simulink model "HvacActrPosnReqFrnt"  (R2022b) (continued)
== system HvacActrPosnReqFrnt == (continued)
  AirFlapFrntStsForRowFirstForActrVentSideLe_AirFlapFrntStsForRowFirstForActrVentSideLe/1 -> HvacActrPosnReqFrnt_Runnable_sys/12(AirFlapFrntStsForRowFirstForActrVentSideLe_AirFlapFrntStsForRowFirstForActrVentSideLe_read)
  AirFlapFrntStsForRowFirstForActrVentSideRi_AirFlapFrntStsForRowFirstForActrVentSideRi/1 -> HvacActrPosnReqFrnt_Runnable_sys/13(AirFlapFrntStsForRowFirstForActrVentSideRi_AirFlapFrntStsForRowFirstForActrVentSideRi_read)
  AirFlapFrntStsForRowFirstForOsa_AirFlapFrntStsForRowFirstForOsa/1 -> HvacActrPosnReqFrnt_Runnable_sys/14(AirFlapFrntStsForRowFirstForOsa_AirFlapFrntStsForRowFirstForOsa_read)
  AirFlapFrntStsForRowFirstForOsaAndRecirc_AirFlapFrntStsForRowFirstForOsaAndRecirc/1 -> HvacActrPosnReqFrnt_Runnable_sys/15(AirFlapFrntStsForRowFirstForOsaAndRecirc_AirFlapFrntStsForRowFirstForOsaAndRecirc_read)
  AirFlapFrntStsForRowFirstForRecirc_AirFlapFrntStsForRowFirstForRecirc/1 -> HvacActrPosnReqFrnt_Runnable_sys/16(AirFlapFrntStsForRowFirstForRecirc_AirFlapFrntStsForRowFirstForRecirc_read)
  AirFlapFrntStsForRowSecForActrFlr_AirFlapFrntStsForRowSecForActrFlr/1 -> HvacActrPosnReqFrnt_Runnable_sys/17(AirFlapFrntStsForRowSecForActrFlr_AirFlapFrntStsForRowSecForActrFlr_read)
  AirFlapFrntStsForRowSecForActrVent_AirFlapFrntStsForRowSecForActrVent/1 -> HvacActrPosnReqFrnt_Runnable_sys/18(AirFlapFrntStsForRowSecForActrVent_AirFlapFrntStsForRowSecForActrVent_read)
  AirFlapPosnFrntActForRowFirstForRecirc_AirFlapPosnFrntActForRowFirstForRecirc/1 -> HvacActrPosnReqFrnt_Runnable_sys/19(AirFlapPosnFrntActForRowFirstForRecirc_AirFlapPosnFrntActForRowFirstForRecirc_read)
  AirFlapSpdActForAirDistbn_AirFlapSpdActForAirDistbn/1 -> HvacActrPosnReqFrnt_Runnable_sys/20(AirFlapSpdActForAirDistbn_AirFlapSpdActForAirDistbn_read)
  AirFlapSpdActForRecirc_AirFlapSpdActForRecirc/1 -> HvacActrPosnReqFrnt_Runnable_sys/21(AirFlapSpdActForRecirc_AirFlapSpdActForRecirc_read)
  AirFlapSpdActForTFrnt_AirFlapSpdActForTFrnt/1 -> HvacActrPosnReqFrnt_Runnable_sys/22(AirFlapSpdActForTFrnt_AirFlapSpdActForTFrnt_read)
  AmbTFildForClimaNgcc_AmbTFildForClima/1 -> HvacActrPosnReqFrnt_Runnable_sys/23(AmbTFildForClimaNgcc_AmbTFildForClima_read)
  AmprFanBoostVentnCnsReIntLe_AmprFanBoostVentnCnsReIntLe/1 -> HvacActrPosnReqFrnt_Runnable_sys/24(AmprFanBoostVentnCnsReIntLe_AmprFanBoostVentnCnsReIntLe_read)
  AmprFanBoostVentnCnsReIntRi_AmprFanBoostVentnCnsReIntRi/1 -> HvacActrPosnReqFrnt_Runnable_sys/25(AmprFanBoostVentnCnsReIntRi_AmprFanBoostVentnCnsReIntRi_read)
  BlwAftRunReq_BlwAftRunReq/1 -> HvacActrPosnReqFrnt_Runnable_sys/26(BlwAftRunReq_BlwAftRunReq_read)
  BlwAftRunSts_BlwAftRunSts/1 -> HvacActrPosnReqFrnt_Runnable_sys/27(BlwAftRunSts_BlwAftRunSts_read)
  ClimaCfgForActrModVrnt1_ClimaCfgForActrInd1/1 -> HvacActrPosnReqFrnt_Runnable_sys/28(ClimaCfgForActrModVrnt1_ClimaCfgForActrInd1_read)
  ClimaCfgForActrModVrnt1_ClimaCfgForActrModVrnt1/1 -> HvacActrPosnReqFrnt_Runnable_sys/29(ClimaCfgForActrModVrnt1_ClimaCfgForActrModVrnt1_read)
  ClimaCfgForActrModVrnt1_ClimaCfgForActrModVrntCen/1 -> HvacActrPosnReqFrnt_Runnable_sys/30(ClimaCfgForActrModVrnt1_ClimaCfgForActrModVrntCen_read)
  ClimaCfgForActrModVrnt2_ClimaCfgForActrInd2/1 -> HvacActrPosnReqFrnt_Runnable_sys/31(ClimaCfgForActrModVrnt2_ClimaCfgForActrInd2_read)
  ClimaCfgForActrModVrnt2_ClimaCfgForActrModVrnt2/1 -> HvacActrPosnReqFrnt_Runnable_sys/32(ClimaCfgForActrModVrnt2_ClimaCfgForActrModVrnt2_read)
  ClimaCfgForActrRecircAndOsaCmnPrsnt1_ClimaCfgForActrRecircAndOsaCmnPrsnt1/1 -> HvacActrPosnReqFrnt_Runnable_sys/33(ClimaCfgForActrRecircAndOsaCmnPrsnt1_ClimaCfgForActrRecircAndOsaCmnPrsnt1_read)
  ClimaCfgForBlowrInRowSecVentSidePrsnt1_ClimaCfgForBlowrInRowSecVentSidePrsnt1/1 -> HvacActrPosnReqFrnt_Runnable_sys/34(ClimaCfgForBlowrInRowSecVentSidePrsnt1_ClimaCfgForBlowrInRowSecVentSidePrsnt1_read)
  ClimaCfgForCarTyp1_ClimaCfgForCarTyp1/1 -> HvacActrPosnReqFrnt_Runnable_sys/35(ClimaCfgForCarTyp1_ClimaCfgForCarTyp1_read)
  ClimaCfgForClimaCtrlMan1_ClimaCfgForClimaCtrlMan1/1 -> HvacActrPosnReqFrnt_Runnable_sys/36(ClimaCfgForClimaCtrlMan1_ClimaCfgForClimaCtrlMan1_read)
  ClimaCfgForExtVentnClimaCtrl_ClimaCfgForExtVentnClimaCtrl/1 -> HvacActrPosnReqFrnt_Runnable_sys/37(ClimaCfgForExtVentnClimaCtrl_ClimaCfgForExtVentnClimaCtrl_read)
  ClimaCfgForFanPotiCtrld1_ClimaCfgForFanPotiCtrld1/1 -> HvacActrPosnReqFrnt_Runnable_sys/38(ClimaCfgForFanPotiCtrld1_ClimaCfgForFanPotiCtrld1_read)
  ClimaCfgForHvacFrontBlowerType_ClimaCfgForHvacFrontBlowerType/1 -> HvacActrPosnReqFrnt_Runnable_sys/39(ClimaCfgForHvacFrontBlowerType_ClimaCfgForHvacFrontBlowerType_read)
  ClimaCfgForVehElec1_ClimaCfgForVehElec1/1 -> HvacActrPosnReqFrnt_Runnable_sys/40(ClimaCfgForVehElec1_ClimaCfgForVehElec1_read)
  ClimaCfgForVrntOfVehSysElec1_ClimaCfgForVrntOfVehSysElec1/1 -> HvacActrPosnReqFrnt_Runnable_sys/41(ClimaCfgForVrntOfVehSysElec1_ClimaCfgForVrntOfVehSysElec1_read)
  ClimaClsDwn_ClimaClsDwn/1 -> HvacActrPosnReqFrnt_Runnable_sys/42(ClimaClsDwn_ClimaClsDwn_read)
  ClimaCtrlActvNgcc_ClimaCtrlActv/1 -> HvacActrPosnReqFrnt_Runnable_sys/43(ClimaCtrlActvNgcc_ClimaCtrlActv_read)
  CmptmtClngActv_CmptmtClngActv/1 -> HvacActrPosnReqFrnt_Runnable_sys/44(CmptmtClngActv_CmptmtClngActv_read)
  CoolgReqForDispActv_CoolgReqForDispActv/1 -> HvacActrPosnReqFrnt_Runnable_sys/45(CoolgReqForDispActv_CoolgReqForDispActv_read)
  ExtVentStsFrmClima_ExtVentStsFrmClima/1 -> HvacActrPosnReqFrnt_Runnable_sys/46(ExtVentStsFrmClima_ExtVentStsFrmClima_read)
  FlowRednAtPwrLvlLo_FlowRednAtPwrLvlLo/1 -> HvacActrPosnReqFrnt_Runnable_sys/47(FlowRednAtPwrLvlLo_FlowRednAtPwrLvlLo_read)
  FogFlashPrevnActv_FogFlashPrevnActv/1 -> HvacActrPosnReqFrnt_Runnable_sys/48(FogFlashPrevnActv_FogFlashPrevnActv_read)
  FrntHvacBlowerSts_FrntHvacBlowerSts/1 -> HvacActrPosnReqFrnt_Runnable_sys/49(FrntHvacBlowerSts_FrntHvacBlowerSts_read)
  HmiCmptmtAirDistbnFrntForClima_HmiCmptmtAirDistbnFrntForClima/1 -> HvacActrPosnReqFrnt_Runnable_sys/50(HmiCmptmtAirDistbnFrntForClima_HmiCmptmtAirDistbnFrntForClima_read)
  HmiCmptmtAirDistbnFrntForClima_HmiCmptmtAirDistbnFrntRiForClima/1 -> HvacActrPosnReqFrnt_Runnable_sys/51(HmiCmptmtAirDistbnFrntForClima_HmiCmptmtAirDistbnFrntRiForClima_read)
  HmiCmptmtAirDistbnFrntForClima_HmiCmptmtAirDistbnReForClima/1 -> HvacActrPosnReqFrnt_Runnable_sys/52(HmiCmptmtAirDistbnFrntForClima_HmiCmptmtAirDistbnReForClima_read)
  HmiCmptmtAirDistbnFrntForClima_HmiCmptmtAirDistbnReRiForClima/1 -> HvacActrPosnReqFrnt_Runnable_sys/53(HmiCmptmtAirDistbnFrntForClima_HmiCmptmtAirDistbnReRiForClima_read)
  HvacCtrlrFanBoostReqLe_HvacCtrlrFanBoostReqLe/1 -> HvacActrPosnReqFrnt_Runnable_sys/54(HvacCtrlrFanBoostReqLe_HvacCtrlrFanBoostReqLe_read)
  HvacCtrlrFanBoostReqRi_HvacCtrlrFanBoostReqRi/1 -> HvacActrPosnReqFrnt_Runnable_sys/55(HvacCtrlrFanBoostReqRi_HvacCtrlrFanBoostReqRi_read)
  HvacFanIFrnt_HvacFanIFrnt/1 -> HvacActrPosnReqFrnt_Runnable_sys/56(HvacFanIFrnt_HvacFanIFrnt_read)
  HvacFanSpdFrnt_HvacFanSpdFrnt/1 -> HvacActrPosnReqFrnt_Runnable_sys/57(HvacFanSpdFrnt_HvacFanSpdFrnt_read)
  HvacFildReqFrntForFan_HvacFildReqFrntForFan/1 -> HvacActrPosnReqFrnt_Runnable_sys/58(HvacFildReqFrntForFan_HvacFildReqFrntForFan_read)
  HvacFildReqFrntForRowFirstForActrDefrst_HvacFildReqFrntForRowFirstForActrDefrst/1 -> HvacActrPosnReqFrnt_Runnable_sys/59(HvacFildReqFrntForRowFirstForActrDefrst_HvacFildReqFrntForRowFirstForActrDefrst_read)
  HvacFildReqFrntForRowFirstForActrFlr_HvacFildReqFrntForRowFirstForActrFlr/1 -> HvacActrPosnReqFrnt_Runnable_sys/60(HvacFildReqFrntForRowFirstForActrFlr_HvacFildReqFrntForRowFirstForActrFlr_read)
  HvacFildReqFrntForRowFirstForActrFlr_HvacFildReqFrntForRowFirstForActrFlrLe/1 -> HvacActrPosnReqFrnt_Runnable_sys/61(HvacFildReqFrntForRowFirstForActrFlr_HvacFildReqFrntForRowFirstForActrFlrLe_read)
  HvacFildReqFrntForRowFirstForActrFlr_HvacFildReqFrntForRowFirstForActrFlrRi/1 -> HvacActrPosnReqFrnt_Runnable_sys/62(HvacFildReqFrntForRowFirstForActrFlr_HvacFildReqFrntForRowFirstForActrFlrRi_read)
  HvacFildReqFrntForRowFirstForActrMod_HvacFildReqFrntForRowFirstForActrMod/1 -> HvacActrPosnReqFrnt_Runnable_sys/63(HvacFildReqFrntForRowFirstForActrMod_HvacFildReqFrntForRowFirstForActrMod_read)
  HvacFildReqFrntForRowFirstForActrTLe_HvacFildReqFrntForRowFirstForActrTLe/1 -> HvacActrPosnReqFrnt_Runnable_sys/64(HvacFildReqFrntForRowFirstForActrTLe_HvacFildReqFrntForRowFirstForActrTLe_read)
  HvacFildReqFrntForRowFirstForActrTRi_HvacFildReqFrntForRowFirstForActrTRi/1 -> HvacActrPosnReqFrnt_Runnable_sys/65(HvacFildReqFrntForRowFirstForActrTRi_HvacFildReqFrntForRowFirstForActrTRi_read)
  HvacFildReqFrntForRowFirstForActrVent_HvacFildReqFrntForRowFirstForActrVent/1 -> HvacActrPosnReqFrnt_Runnable_sys/66(HvacFildReqFrntForRowFirstForActrVent_HvacFildReqFrntForRowFirstForActrVent_read)
  HvacFildReqFrntForRowFirstForActrVent_HvacFildReqFrntForRowFirstForActrVentLe/1 -> HvacActrPosnReqFrnt_Runnable_sys/67(HvacFildReqFrntForRowFirstForActrVent_HvacFildReqFrntForRowFirstForActrVentLe_read)
  HvacFildReqFrntForRowFirstForActrVent_HvacFildReqFrntForRowFirstForActrVentRi/1 -> HvacActrPosnReqFrnt_Runnable_sys/68(HvacFildReqFrntForRowFirstForActrVent_HvacFildReqFrntForRowFirstForActrVentRi_read)
  HvacFildReqFrntForRowFirstForActrVent_HvacFildReqFrntForRowFirstForActrVentSideLe/1 -> HvacActrPosnReqFrnt_Runnable_sys/69(HvacFildReqFrntForRowFirstForActrVent_HvacFildReqFrntForRowFirstForActrVentSideLe_read)
  HvacFildReqFrntForRowFirstForActrVent_HvacFildReqFrntForRowFirstForActrVentSideRi/1 -> HvacActrPosnReqFrnt_Runnable_sys/70(HvacFildReqFrntForRowFirstForActrVent_HvacFildReqFrntForRowFirstForActrVentSideRi_read)
  HvacFildReqFrntForRowFirstForOsa_HvacFildReqFrntForRowFirstForOsa/1 -> HvacActrPosnReqFrnt_Runnable_sys/71(HvacFildReqFrntForRowFirstForOsa_HvacFildReqFrntForRowFirstForOsa_read)
  HvacFildReqFrntForRowFirstForOsaAndRecirc_HvacFildReqFrntForRowFirstForOsaAndRecirc/1 -> HvacActrPosnReqFrnt_Runnable_sys/72(HvacFildReqFrntForRowFirstForOsaAndRecirc_HvacFildReqFrntForRowFirstForOsaAndRecirc_read)
  HvacFildReqFrntForRowFirstForRecirc_HvacFildReqFrntForRowFirstForRecirc/1 -> HvacActrPosnReqFrnt_Runnable_sys/73(HvacFildReqFrntForRowFirstForRecirc_HvacFildReqFrntForRowFirstForRecirc_read)
  HvacFildReqFrntForRowFirstLeForActrMod_HvacFildReqFrntForRowFirstLeForActrMod/1 -> HvacActrPosnReqFrnt_Runnable_sys/74(HvacFildReqFrntForRowFirstLeForActrMod_HvacFildReqFrntForRowFirstLeForActrMod_read)
  HvacFildReqFrntForRowSecForActrFlr_HvacFildReqFrntForRowSecForActrFlr/1 -> HvacActrPosnReqFrnt_Runnable_sys/75(HvacFildReqFrntForRowSecForActrFlr_HvacFildReqFrntForRowSecForActrFlr_read)
  HvacFildReqFrntForRowSecForActrModLe_HvacFildReqFrntForRowSecForActrModLe/1 -> HvacActrPosnReqFrnt_Runnable_sys/76(HvacFildReqFrntForRowSecForActrModLe_HvacFildReqFrntForRowSecForActrModLe_read)
  HvacFildReqFrntForRowSecForActrModRi_HvacFildReqFrntForRowSecForActrModRi/1 -> HvacActrPosnReqFrnt_Runnable_sys/77(HvacFildReqFrntForRowSecForActrModRi_HvacFildReqFrntForRowSecForActrModRi_read)
  HvacFildReqFrntForRowSecForActrTLe_HvacFildReqFrntForRowSecForActrTLe/1 -> HvacActrPosnReqFrnt_Runnable_sys/78(HvacFildReqFrntForRowSecForActrTLe_HvacFildReqFrntForRowSecForActrTLe_read)
  HvacFildReqFrntForRowSecForActrTRi_HvacFildReqFrntForRowSecForActrTRi/1 -> HvacActrPosnReqFrnt_Runnable_sys/79(HvacFildReqFrntForRowSecForActrTRi_HvacFildReqFrntForRowSecForActrTRi_read)
  HvacFildReqFrntForRowSecForActrVent_HvacFildReqFrntForRowSecForActrVent/1 -> HvacActrPosnReqFrnt_Runnable_sys/80(HvacFildReqFrntForRowSecForActrVent_HvacFildReqFrntForRowSecForActrVent_read)
  HvacRecircRatReq_HvacRecircRatReq/1 -> HvacActrPosnReqFrnt_Runnable_sys/81(HvacRecircRatReq_HvacRecircRatReq_read)
  HvacTarFlowTot_HvacTarFlowTot/1 -> HvacActrPosnReqFrnt_Runnable_sys/82(HvacTarFlowTot_HvacTarFlowTot_read)
  IntCo2ActvSts_IntCo2ActvSts/1 -> HvacActrPosnReqFrnt_Runnable_sys/83(IntCo2ActvSts_IntCo2ActvSts_read)
  InteCleanUnpleSmell_Ngcc_InteCleanUnpleSmell/1 -> HvacActrPosnReqFrnt_Runnable_sys/84(InteCleanUnpleSmell_Ngcc_InteCleanUnpleSmell_read)
  MemReadForHvacActrPosnReqFrnt_MemReadForHvacActrPosnReqFrnt/1 -> HvacActrPosnReqFrnt_Runnable_sys/85(MemReadForHvacActrPosnReqFrnt_MemReadForHvacActrPosnReqFrnt_read)
  MemReqStsForHvac_MemReqStsForHvac/1 -> HvacActrPosnReqFrnt_Runnable_sys/86(MemReqStsForHvac_MemReqStsForHvac_read)
  PrkgClimaActv_PrkgClimaActv/1 -> HvacActrPosnReqFrnt_Runnable_sys/87(PrkgClimaActv_PrkgClimaActv_read)
  PwrAllwdForHvacFanFrnt_PwrAllwdForHvacFanFrnt/1 -> HvacActrPosnReqFrnt_Runnable_sys/88(PwrAllwdForHvacFanFrnt_PwrAllwdForHvacFanFrnt_read)
  RemVentActvSts_RemVentActvSts/1 -> HvacActrPosnReqFrnt_Runnable_sys/89(RemVentActvSts_RemVentActvSts_read)
  SplyUForClimaCtrl_SplyUForClimaCtrl/1 -> HvacActrPosnReqFrnt_Runnable_sys/90(SplyUForClimaCtrl_SplyUForClimaCtrl_read)
  TWinRfClsdPopUpReq_TWinRfClsdPopUpReq/1 -> HvacActrPosnReqFrnt_Runnable_sys/91(TWinRfClsdPopUpReq_TWinRfClsdPopUpReq_read)
  VehModMngtGlbSafe1_Ngcc_VehModMngtGlbSafe1/1 -> HvacActrPosnReqFrnt_Runnable_sys/92(VehModMngtGlbSafe1_Ngcc_VehModMngtGlbSafe1_read)
  VehSpdLgtForClima_VehSpdLgtForClima/1 -> HvacActrPosnReqFrnt_Runnable_sys/93(VehSpdLgtForClima_VehSpdLgtForClima_read)
  VentnForWinOpenGlbActv_VentnForWinOpenGlbActv/1 -> HvacActrPosnReqFrnt_Runnable_sys/94(VentnForWinOpenGlbActv_VentnForWinOpenGlbActv_read)
  VoltFanBoostVentnMeasReIntLe_VoltFanBoostVentnMeasReIntLe/1 -> HvacActrPosnReqFrnt_Runnable_sys/95(VoltFanBoostVentnMeasReIntLe_VoltFanBoostVentnMeasReIntLe_read)
  VoltFanBoostVentnMeasReIntRi_VoltFanBoostVentnMeasReIntRi/1 -> HvacActrPosnReqFrnt_Runnable_sys/96(VoltFanBoostVentnMeasReIntRi_VoltFanBoostVentnMeasReIntRi_read)
  WinRfClsdReqForCoolgDwn_WinRfClsdReqForCoolgDwn/1 -> HvacActrPosnReqFrnt_Runnable_sys/97(WinRfClsdReqForCoolgDwn_WinRfClsdReqForCoolgDwn_read)
  AirFlapFrntStsForRowFirstModDistbnLe_AirFlapFrntStsForRowFirstModDistbnLe/1 -> HvacActrPosnReqFrnt_Runnable_sys/98(AirFlapFrntStsForRowFirstModDistbnLe_AirFlapFrntStsForRowFirstModDistbnLe_read)
  AirFlowLimDurgMidAmbtForNVHForHVACFrntFlag_AirFlowLimDurgMidAmbtForNVHForHVACFrntFlag/1 -> HvacActrPosnReqFrnt_Runnable_sys/99(AirFlowLimDurgMidAmbtForNVHForHVACFrntFlag_AirFlowLimDurgMidAmbtForNVHForHVACFrntFlag_read)
  AmbTFildForClimaQf_AmbTFildForClimaQf/1 -> HvacActrPosnReqFrnt_Runnable_sys/100(AmbTFildForClimaQf_AmbTFildForClimaQf_read)
  ClimaCfgReqVersionReplace_ClimaCfgReqVersionReplace/1 -> HvacActrPosnReqFrnt_Runnable_sys/101(ClimaCfgReqVersionReplace_ClimaCfgReqVersionReplace_read)
  FrntBlwWrkLmt_FrntBlwWrkLmt/1 -> HvacActrPosnReqFrnt_Runnable_sys/102(FrntBlwWrkLmt_FrntBlwWrkLmt_read)
  FrntClimaManModCmftStsNgcc_FrntClimaManModCmftStsNgcc/1 -> HvacActrPosnReqFrnt_Runnable_sys/103(FrntClimaManModCmftStsNgcc_FrntClimaManModCmftStsNgcc_read)
  HighTempCleanRunningStsNgcc_HighTempCleanRunningStsNgcc/1 -> HvacActrPosnReqFrnt_Runnable_sys/104(HighTempCleanRunningStsNgcc_HighTempCleanRunningStsNgcc_read)
  HighTempClnActv_HighTempClnActv/1 -> HvacActrPosnReqFrnt_Runnable_sys/105(HighTempClnActv_HighTempClnActv_read)
  HmiCarLockACRecircSetNgcc_HmiCarLockACRecircSetNgcc/1 -> HvacActrPosnReqFrnt_Runnable_sys/106(HmiCarLockACRecircSetNgcc_HmiCarLockACRecircSetNgcc_read)
  HmiDefrstMaxReqForClima_HmiDefrstMaxReqForClima/1 -> HvacActrPosnReqFrnt_Runnable_sys/107(HmiDefrstMaxReqForClima_HmiDefrstMaxReqForClima_read)
  HmiHvacFanLvlFrntForClima_HmiHvacFanLvlFrntForClima/1 -> HvacActrPosnReqFrnt_Runnable_sys/108(HmiHvacFanLvlFrntForClima_HmiHvacFanLvlFrntForClima_read)
  HmiHvacFanLvlFrntForClima_HmiHvacFanLvlFrntRiForClima/1 -> HvacActrPosnReqFrnt_Runnable_sys/109(HmiHvacFanLvlFrntForClima_HmiHvacFanLvlFrntRiForClima_read)
  HmiMaxACReqForClima_HmiMaxACReqForClima/1 -> HvacActrPosnReqFrnt_Runnable_sys/110(HmiMaxACReqForClima_HmiMaxACReqForClima_read)
  HvacPwm2MosVFbFrnt_HvacPwm2MosVFbFrnt/1 -> HvacActrPosnReqFrnt_Runnable_sys/111(HvacPwm2MosVFbFrnt_HvacPwm2MosVFbFrnt_read)
  HvacPwmNFbFrnt_HvacPwmNFbFrnt/1 -> HvacActrPosnReqFrnt_Runnable_sys/112(HvacPwmNFbFrnt_HvacPwmNFbFrnt_read)
  HvBattLimnIndcnNgcc_HvBattLimnIndcnNgcc/1 -> HvacActrPosnReqFrnt_Runnable_sys/113(HvBattLimnIndcnNgcc_HvBattLimnIndcnNgcc_read)
  LdAdVal_CurrAdVal/1 -> HvacActrPosnReqFrnt_Runnable_sys/114(LdAdVal_CurrAdVal_read)
  LdAdVal_ValidAdVal/1 -> HvacActrPosnReqFrnt_Runnable_sys/115(LdAdVal_ValidAdVal_read)
  ManModeForRowFirstLeForModelDial_ManModeForRowFirstLeForModelDial/1 -> HvacActrPosnReqFrnt_Runnable_sys/116(ManModeForRowFirstLeForModelDial_ManModeForRowFirstLeForModelDial_read)
  MemReadForPrkgRecirc_MemReadForPrkgRecirc/1 -> HvacActrPosnReqFrnt_Runnable_sys/117(MemReadForPrkgRecirc_MemReadForPrkgRecirc_read)
  PrkgClimaOnReq_PrkgClimaOnReq/1 -> HvacActrPosnReqFrnt_Runnable_sys/118(PrkgClimaOnReq_PrkgClimaOnReq_read)
  PrvntWinFrz_PrvntWinFrz/1 -> HvacActrPosnReqFrnt_Runnable_sys/119(PrvntWinFrz_PrvntWinFrz_read)
  HvacActrPosnReqFrnt_Runnable_sys/1(HvacFanLvlFbNgcc_HvacFanLvlFbNgcc_write) -> HvacFanLvlFbNgcc_HvacFanLvlFbNgcc/1
  HvacActrPosnReqFrnt_Runnable_sys/2(HvacFanVoltReqFrnt_HvacFanVoltReqFrnt_write) -> HvacFanVoltReqFrnt_HvacFanVoltReqFrnt/1
  HvacActrPosnReqFrnt_Runnable_sys/3(MemSaveFlgForPrkgRecirc_MemSaveFlgForPrkgRecirc_write) -> MemSaveFlgForPrkgRecirc_MemSaveFlgForPrkgRecirc/1
  HvacActrPosnReqFrnt_Runnable_sys/4(MemWrForPrkgRecirc_MemWrForPrkgRecirc_write) -> MemWrForPrkgRecirc_MemWrForPrkgRecirc/1
  HvacActrPosnReqFrnt_Runnable_sys/5(ActrFrntReqForRowFirstLeModDistbn_ActrFrntReqForRowFirstLeModDistbn_write) -> ActrFrntReqForRowFirstLeModDistbn_ActrFrntReqForRowFirstLeModDistbn/1
  HvacActrPosnReqFrnt_Runnable_sys/6(ActrFrntReqForRowFirstModDistbn_ActrFrntReqForRowFirstModDistbn_write) -> ActrFrntReqForRowFirstModDistbn_ActrFrntReqForRowFirstModDistbn/1
  HvacActrPosnReqFrnt_Runnable_sys/7(ActrFrntReqForRowSecModDistbnLe_ActrFrntReqForRowSecModDistbnLe_write) -> ActrFrntReqForRowSecModDistbnLe_ActrFrntReqForRowSecModDistbnLe/1
  HvacActrPosnReqFrnt_Runnable_sys/8(ActrFrntReqForRowSecModDistbnRi_ActrFrntReqForRowSecModDistbnRi_write) -> ActrFrntReqForRowSecModDistbnRi_ActrFrntReqForRowSecModDistbnRi/1
  HvacActrPosnReqFrnt_Runnable_sys/9(ActrPosnFrnReqForOsaAndRecirc_ActrPosnFrnReqForOsaAndRecirc_write) -> ActrPosnFrnReqForOsaAndRecirc_ActrPosnFrnReqForOsaAndRecirc/1
  HvacActrPosnReqFrnt_Runnable_sys/10(AirFlapPosnFrntReqForRowFirstForActrDefrst_AirFlapPosnFrntReqForRowFirstForActrDefrst_write) -> AirFlapPosnFrntReqForRowFirstForActrDefrst_AirFlapPosnFrntReqForRowFirstForActrDefrst/1
  HvacActrPosnReqFrnt_Runnable_sys/11(AirFlapPosnFrntReqForRowFirstForActrFlr_AirFlapPosnFrntReqForRowFirstForActrFlr_write) -> AirFlapPosnFrntReqForRowFirstForActrFlr_AirFlapPosnFrntReqForRowFirstForActrFlr/1
  HvacActrPosnReqFrnt_Runnable_sys/12(AirFlapPosnFrntReqForRowFirstForActrFlrLe_AirFlapPosnFrntReqForRowFirstForActrFlrLe_write) -> AirFlapPosnFrntReqForRowFirstForActrFlrLe_AirFlapPosnFrntReqForRowFirstForActrFlrLe/1
  HvacActrPosnReqFrnt_Runnable_sys/13(AirFlapPosnFrntReqForRowFirstForActrFlrRi_AirFlapPosnFrntReqForRowFirstForActrFlrRi_write) -> AirFlapPosnFrntReqForRowFirstForActrFlrRi_AirFlapPosnFrntReqForRowFirstForActrFlrRi/1
  HvacActrPosnReqFrnt_Runnable_sys/14(AirFlapPosnFrntReqForRowFirstForActrTLe_AirFlapPosnFrntReqForRowFirstForActrTLe_write) -> AirFlapPosnFrntReqForRowFirstForActrTLe_AirFlapPosnFrntReqForRowFirstForActrTLe/1
  HvacActrPosnReqFrnt_Runnable_sys/15(AirFlapPosnFrntReqForRowFirstForActrTRi_AirFlapPosnFrntReqForRowFirstForActrTRi_write) -> AirFlapPosnFrntReqForRowFirstForActrTRi_AirFlapPosnFrntReqForRowFirstForActrTRi/1
  HvacActrPosnReqFrnt_Runnable_sys/16(AirFlapPosnFrntReqForRowFirstForActrVent_AirFlapPosnFrntReqForRowFirstForActrVent_write) -> AirFlapPosnFrntReqForRowFirstForActrVent_AirFlapPosnFrntReqForRowFirstForActrVent/1
  HvacActrPosnReqFrnt_Runnable_sys/17(AirFlapPosnFrntReqForRowFirstForActrVentLe_AirFlapPosnFrntReqForRowFirstForActrVentLe_write) -> AirFlapPosnFrntReqForRowFirstForActrVentLe_AirFlapPosnFrntReqForRowFirstForActrVentLe/1
  HvacActrPosnReqFrnt_Runnable_sys/18(AirFlapPosnFrntReqForRowFirstForActrVentRi_AirFlapPosnFrntReqForRowFirstForActrVentRi_write) -> AirFlapPosnFrntReqForRowFirstForActrVentRi_AirFlapPosnFrntReqForRowFirstForActrVentRi/1
  HvacActrPosnReqFrnt_Runnable_sys/19(AirFlapPosnFrntReqForRowFirstForActrVentSideLe_AirFlapPosnFrntReqForRowFirstForActrVentSideLe_write) -> AirFlapPosnFrntReqForRowFirstForActrVentSideLe_AirFlapPosnFrntReqForRowFirstForActrVentSideLe/1
  HvacActrPosnReqFrnt_Runnable_sys/20(AirFlapPosnFrntReqForRowFirstForActrVentSideRi_AirFlapPosnFrntReqForRowFirstForActrVentSideRi_write) -> AirFlapPosnFrntReqForRowFirstForActrVentSideRi_AirFlapPosnFrntReqForRowFirstForActrVentSideRi/1
  HvacActrPosnReqFrnt_Runnable_sys/21(AirFlapPosnFrntReqForRowFirstForOsa_AirFlapPosnFrntReqForRowFirstForOsa_write) -> AirFlapPosnFrntReqForRowFirstForOsa_AirFlapPosnFrntReqForRowFirstForOsa/1
  HvacActrPosnReqFrnt_Runnable_sys/22(AirFlapPosnFrntReqForRowFirstForRecirc_AirFlapPosnFrntReqForRowFirstForRecirc_write) -> AirFlapPosnFrntReqForRowFirstForRecirc_AirFlapPosnFrntReqForRowFirstForRecirc/1
  HvacActrPosnReqFrnt_Runnable_sys/23(AirFlapPosnFrntReqForRowSecForActrFlr_AirFlapPosnFrntReqForRowSecForActrFlr_write) -> AirFlapPosnFrntReqForRowSecForActrFlr_AirFlapPosnFrntReqForRowSecForActrFlr/1
  HvacActrPosnReqFrnt_Runnable_sys/24(AirFlapPosnFrntReqForRowSecForActrTLe_AirFlapPosnFrntReqForRowSecForActrTLe_write) -> AirFlapPosnFrntReqForRowSecForActrTLe_AirFlapPosnFrntReqForRowSecForActrTLe/1
  HvacActrPosnReqFrnt_Runnable_sys/25(AirFlapPosnFrntReqForRowSecForActrTRi_AirFlapPosnFrntReqForRowSecForActrTRi_write) -> AirFlapPosnFrntReqForRowSecForActrTRi_AirFlapPosnFrntReqForRowSecForActrTRi/1
  HvacActrPosnReqFrnt_Runnable_sys/26(AirFlapPosnFrntReqForRowSecForActrVent_AirFlapPosnFrntReqForRowSecForActrVent_write) -> AirFlapPosnFrntReqForRowSecForActrVent_AirFlapPosnFrntReqForRowSecForActrVent/1
  HvacActrPosnReqFrnt_Runnable_sys/27(AirFlapSpdReqForAirDistbn_AirFlapSpdReqForAirDistbn_write) -> AirFlapSpdReqForAirDistbn_AirFlapSpdReqForAirDistbn/1
  HvacActrPosnReqFrnt_Runnable_sys/28(AirFlapSpdReqForRecirc_AirFlapSpdReqForRecirc_write) -> AirFlapSpdReqForRecirc_AirFlapSpdReqForRecirc/1
  HvacActrPosnReqFrnt_Runnable_sys/29(AirFlapSpdReqForTFrnt_AirFlapSpdReqForTFrnt_write) -> AirFlapSpdReqForTFrnt_AirFlapSpdReqForTFrnt/1
  HvacActrPosnReqFrnt_Runnable_sys/30(ClimaCtrlVFCClnCmptmtClima_ClimaCtrlVFCClnCmptmtClima_write) -> ClimaCtrlVFCClnCmptmtClima_ClimaCtrlVFCClnCmptmtClima/1
  FanBoostVentnRampReIntLe_FanBoostVentnRampReIntLe_merge/1 -> FanBoostVentnRampReIntLe_FanBoostVentnRampReIntLe/1
  FanBoostVentnRampReIntRi_FanBoostVentnRampReIntRi_merge/1 -> FanBoostVentnRampReIntRi_FanBoostVentnRampReIntRi/1
  HvacActrPosnReqFrnt_Runnable_sys/33(FanBoostVentnSpReqReIntLe_FanBoostVentnSpReqReIntLe_write) -> FanBoostVentnSpReqReIntLe_FanBoostVentnSpReqReIntLe/1
  HvacActrPosnReqFrnt_Runnable_sys/34(FanBoostVentnSpReqReIntRi_FanBoostVentnSpReqReIntRi_write) -> FanBoostVentnSpReqReIntRi_FanBoostVentnSpReqReIntRi/1
  HvacActrPosnReqFrnt_Runnable_sys/35(HvacFanDutyReqFrnt_HvacFanDutyReqFrnt_write) -> HvacFanDutyReqFrnt_HvacFanDutyReqFrnt/1
  HvacActrPosnReqFrnt_Runnable_sys/36(HvacFanFrntLimForPwrAllwd_HvacFanFrntLimForPwrAllwd_write) -> HvacFanFrntLimForPwrAllwd_HvacFanFrntLimForPwrAllwd/1
  HvacActrPosnReqFrnt_Runnable_sys/37(HvacFanReqFrnt_HvacFanReqFrnt_write) -> HvacFanReqFrnt_HvacFanReqFrnt/1
  HvacActrPosnReqFrnt_Runnable_sys/38(MemSaveFlgForHvacActrPosnReqFrnt_MemSaveFlgForHvacActrPosnReqFrnt_write) -> MemSaveFlgForHvacActrPosnReqFrnt_MemSaveFlgForHvacActrPosnReqFrnt/1
  HvacActrPosnReqFrnt_Runnable_sys/39(MemWrForHvacActrPosnReqFrnt_MemWrForHvacActrPosnReqFrnt_write) -> MemWrForHvacActrPosnReqFrnt_MemWrForHvacActrPosnReqFrnt/1
  HvacActrPosnReqFrnt_Runnable_sys/40(PwrCnsDesForHvacFanFrnt_PwrCnsDesForHvacFanFrnt_write) -> PwrCnsDesForHvacFanFrnt_PwrCnsDesForHvacFanFrnt/1
  HvacActrPosnReqFrnt_Runnable_sys/41(PwrCnsDurgEngStopForFlapActr_PwrCnsDurgEngStopForFlapActr_write) -> PwrCnsDurgEngStopForFlapActr_PwrCnsDurgEngStopForFlapActr/1
  PwrCnsDurgEngStopForHvacFanFrnt_PwrCnsDurgEngStopForHvacFanFrnt_merge/1 -> PwrCnsDurgEngStopForHvacFanFrnt_PwrCnsDurgEngStopForHvacFanFrnt/1
  HvacActrPosnReqFrnt_Runnable_sys/43(PwrCnsForFlapActr_PwrCnsForFlapActr_write) -> PwrCnsForFlapActr_PwrCnsForFlapActr/1
  PwrCnsForHvacFanFrntNgcc_PwrCnsForHvacFanFrnt_merge/1 -> PwrCnsForHvacFanFrntNgcc_PwrCnsForHvacFanFrnt/1
  ReqForActrCal_ReqForActrCal_merge/1 -> ReqForActrCal_ReqForActrCal/1
  HvacActrPosnReqFrnt_Runnable_sys/45(ReqForActrCal_ReqForActrCal_write) -> [ReqForActrCal_ReqForActrCal_merge_Tag]
  [ReqForActrCal_ReqForActrCal_merge_Tag] -> ReqForActrCal_ReqForActrCal_merge/2
  HvacActrPosnReqFrnt_Init/5(ReqForActrCal_ReqForActrCal_write) -> [ReqForActrCal_ReqForActrCal_merge_Tag1]
  [ReqForActrCal_ReqForActrCal_merge_Tag1] -> ReqForActrCal_ReqForActrCal_merge/1
  HvacActrPosnReqFrnt_Runnable_sys/44(PwrCnsForHvacFanFrntNgcc_PwrCnsForHvacFanFrnt_write) -> [PwrCnsForHvacFanFrntNgcc_PwrCnsForHvacFanFrnt_merge_Tag]
  [PwrCnsForHvacFanFrntNgcc_PwrCnsForHvacFanFrnt_merge_Tag] -> PwrCnsForHvacFanFrntNgcc_PwrCnsForHvacFanFrnt_merge/2
  HvacActrPosnReqFrnt_Init/4(PwrCnsForHvacFanFrntNgcc_PwrCnsForHvacFanFrnt_write) -> [PwrCnsForHvacFanFrntNgcc_PwrCnsForHvacFanFrnt_merge_Tag1]
  [PwrCnsForHvacFanFrntNgcc_PwrCnsForHvacFanFrnt_merge_Tag1] -> PwrCnsForHvacFanFrntNgcc_PwrCnsForHvacFanFrnt_merge/1
  HvacActrPosnReqFrnt_Runnable_sys/42(PwrCnsDurgEngStopForHvacFanFrnt_PwrCnsDurgEngStopForHvacFanFrnt_write) -> [PwrCnsDurgEngStopForHvacFanFrnt_PwrCnsDurgEngS_4ccc3db916fe7762]
  [PwrCnsDurgEngStopForHvacFanFrnt_PwrCnsDurgEngS_4ccc3db916fe7762] -> PwrCnsDurgEngStopForHvacFanFrnt_PwrCnsDurgEngStopForHvacFanFrnt_merge/2
  HvacActrPosnReqFrnt_Init/3(PwrCnsDurgEngStopForHvacFanFrnt_PwrCnsDurgEngStopForHvacFanFrnt_write) -> [PwrCnsDurgEngStopForHvacFanFrnt_PwrCnsDurgEngS_97c37e6b787f9cc2]
  [PwrCnsDurgEngStopForHvacFanFrnt_PwrCnsDurgEngS_97c37e6b787f9cc2] -> PwrCnsDurgEngStopForHvacFanFrnt_PwrCnsDurgEngStopForHvacFanFrnt_merge/1
  HvacActrPosnReqFrnt_Runnable_sys/32(FanBoostVentnRampReIntRi_FanBoostVentnRampReIntRi_write) -> [FanBoostVentnRampReIntRi_FanBoostVentnRampReIntRi_merge_Tag]
  [FanBoostVentnRampReIntRi_FanBoostVentnRampReIntRi_merge_Tag] -> FanBoostVentnRampReIntRi_FanBoostVentnRampReIntRi_merge/2
  HvacActrPosnReqFrnt_Init/2(FanBoostVentnRampReIntRi_FanBoostVentnRampReIntRi_write) -> [FanBoostVentnRampReIntRi_FanBoostVentnRampReIntRi_merge_Tag1]
  [FanBoostVentnRampReIntRi_FanBoostVentnRampReIntRi_merge_Tag1] -> FanBoostVentnRampReIntRi_FanBoostVentnRampReIntRi_merge/1
  HvacActrPosnReqFrnt_Runnable_sys/31(FanBoostVentnRampReIntLe_FanBoostVentnRampReIntLe_write) -> [FanBoostVentnRampReIntLe_FanBoostVentnRampReIntLe_merge_Tag]
  [FanBoostVentnRampReIntLe_FanBoostVentnRampReIntLe_merge_Tag] -> FanBoostVentnRampReIntLe_FanBoostVentnRampReIntLe_merge/2
  HvacActrPosnReqFrnt_Init/1(FanBoostVentnRampReIntLe_FanBoostVentnRampReIntLe_write) -> [FanBoostVentnRampReIntLe_FanBoostVentnRampReIntLe_merge_Tag1]
  [FanBoostVentnRampReIntLe_FanBoostVentnRampReIntLe_merge_Tag1] -> FanBoostVentnRampReIntLe_FanBoostVentnRampReIntLe_merge/1
  HvacActrPosnReqFrnt_Runnable/1 -> HvacActrPosnReqFrnt_Runnable_sys/Trigger
